simulink model "TosAeb"  (R2021a)
settings: Solver=FixedStepDiscrete  StartTime=0.0  StopTime=inf  FixedStep=0.01  RelTol=1e-3
undefined symbols (not defined in the file or in its folder): ACCModSts, AccSts1, AEB_ObjectMovingOrientation, AEB_ObjectMovingState, CN_BTCActive, CN_CurrentGearR, CN_CurrentGearR_DHT, CN_PTCActive, CN_VDCActive, CN_VSC_EspOff, CN_VSC_FCW_AEB_Error, CN_VSC_FCW_AEB_NoDispay, CN_VSC_FCW_AEB_PerfmDgdtn, CN_VSC_FCW_AEB_TempUnAvlbl, CN_VSC_VehStandstill, CNaccelAvoidMax, CNaccelAvoidMin, CNbrakePedalPress, CNcomfortStrLeftAccelMax, CNcomfortStrLeftAccelMin, CNcomfortStrRightAccelMax, CNcomfortStrRightAccelMin, CNDrvDoorOpen, CNDrvModeEco, CNDrvModeNorm, CNDrvModeOther, CNDrvModeSport, CNDrvSBRreminder, CNNull, CNobjInvalidID, CNPassDoorOpen, CNPassSBRreminder, CNPEBSAccelNegInf, CNPEBSAccelNull, CNPEBSTimeInf, CNPEBSTimeNull, CNSTOPMAX, CNSTOPNULL, CNsubjWidth, CNSwtOFF, CNSwtON, CNTTCMAX, CNTTCNULL, CNVehConfigAEBCycNone, CNVehConfigAEBNone, CNVehConfigAEBPedNone, CNVehConfigFCWNone, CNVehConfigJANone, CollisionSeverity, FALSE, FCWSnvty, JACycObjCalcInfoDefaultParams, JAPedObjCalcInfoDefaultParams, JAVehObjCalcInfoDefaultParams, latCycObjCalcInfoDefaultParams, latPedObjCalcInfoDefaultParams, longObjCalcInfoDefaultParams, ModSts, movingStatus, ObjectType ... (+1112)
libraries: simulink x3031, AEBSFunctionLib x50, Ta_Library x33, AEBSStateMachineLib x23, Mylibrary x12, simulink_extras x7, ObjSelect_lib x3, dspstat3 x2
(part 88 of 102)

== system TosAeb/FcwAebJa_Step_sys/ALG_AEBSystem/ALG_AEBController/AEB_LongTgtInfoCalc/LongObjCalcInfoCalc/AEBS_LongCollisionAssesApp/LongCollisionAvoidAssess/HighLvlBrkCalc/HighLvlBrkCalc4Brake/accelAvoidHighLvlBrkSituation3/If Action Subsystem1/accelAvoidHighLvlBrkCalc/accelAvoidLowLvlBrkCalc/Situation3 ==
  note: aEgo^2
      | aAvoid = ----------------------------------
      | 		vObj^2
      | 		---------  - 2(dRel - dScure)
      | 		aObj
  subjVel : Inport
  vxAbsObj : Inport  Port=2
  axAbsObj : Inport  Port=3
  dxEgoBrake : Inport  Port=4
  function : TriggerPort  TriggerType=function-call  VariantControl=Variant
  Divide : Product  Inputs=*/
  Divide1 : Product  Inputs=*/
  Gain : Gain  Gain=2
  Math Function : Math  Operator=square  SignedPower=on  SaturateOnIntegerOverflow=on
  Math Function1 : Math  Operator=square  SignedPower=on  SaturateOnIntegerOverflow=on
  Subtract : Sum  Inputs=+-
  accelAvoidLowLvlBrk : Outport
  wires:
  vxAbsObj/1 -> Math Function/1
  subjVel/1 -> Math Function1/1
  Math Function/1 -> Divide/1
  Gain/1 -> Subtract/2
  Divide1/1 -> accelAvoidLowLvlBrk/1
  Math Function1/1 -> Divide1/1
  Divide/1 -> Subtract/1
  axAbsObj/1 -> Divide/2
  dxEgoBrake/1 -> Gain/1
  Subtract/1 -> Divide1/2

== system TosAeb/FcwAebJa_Step_sys/ALG_AEBSystem/ALG_AEBController/AEB_LongTgtInfoCalc/LongObjCalcInfoCalc/AEBS_LongCollisionAssesApp/LongCollisionAvoidAssess/HighLvlBrkCalc/HighLvlBrkCalc4Brake/accelAvoidHighLvlBrkSituation3/If Action Subsystem1/accelAvoidHighLvlBrkCalc/accelAvoidLowLvlBrkCalc/Situation4 ==
  function : TriggerPort  TriggerType=function-call  VariantControl=Variant
  Constant : Constant  Value=CNNull  VectorParams1D=off
  accelAvoidLowLvlBrk : Outport
  wires:
  Constant/1 -> accelAvoidLowLvlBrk/1

== system TosAeb/FcwAebJa_Step_sys/ALG_AEBSystem/ALG_AEBController/AEB_LongTgtInfoCalc/LongObjCalcInfoCalc/AEBS_LongCollisionAssesApp/LongCollisionAvoidAssess/HighLvlBrkCalc/HighLvlBrkCalc4Brake/accelAvoidHighLvlBrkSituation3/If Action Subsystem1/accelAvoidHighLvlBrkCalc/accelAvoidLowLvlBrkCalc/accelAvoidLowLvlBrkLimit ==
  accelAvoidLowLvlBrkNoLimit : Inport
  Saturation : Saturate  UpperLimit=CNNull  LowerLimit=CNaccelAvoidMin
      settings: AttributesFormatString=|
      | UpperLimit:%<UpperLimit>
      | LowerLimit:%<LowerLimit>
  accelAvoidLowLvlBrk : Outport
  wires:
  accelAvoidLowLvlBrkNoLimit/1 -> Saturation/1
  Saturation/1 -> accelAvoidLowLvlBrk/1

== system TosAeb/FcwAebJa_Step_sys/ALG_AEBSystem/ALG_AEBController/AEB_LongTgtInfoCalc/LongObjCalcInfoCalc/AEBS_LongCollisionAssesApp/LongCollisionAvoidAssess/HighLvlBrkCalc/HighLvlBrkCalc4Brake/accelAvoidHighLvlBrkSituation3/If Action Subsystem1/accelAvoidHighLvlBrkCalc/accelAvoidLowLvlBrkCalc1/Situation1 ==
  dxEgoBrake : Inport
  vx : Inport  Port=2
  tObjStop : Inport  Port=3
  subjVel : Inport  Port=4
  vxAbsObj : Inport  Port=5
  axAbsObj : Inport  Port=6
  function : TriggerPort  TriggerType=function-call  VariantControl=Variant
  Bus Creator : BusCreator  Inputs=6
  Bus Selector : BusSelector  OutputSignals=dxEgoBrake,vxRelTargetObjCalc,tObjStop
  Bus Selector1 : BusSelector  OutputSignals=axAbsTargetObjCalc,vxRelTargetObjCalc,dxEgoBrake
  Bus Selector2 : BusSelector  OutputSignals=subjVel,vxAbsTargetObjCalc,axAbsTargetObjCalc,dxEgoBrake
  Case1 : Subsystem  TreatAsAtomicUnit=on
  Case2 : Subsystem  TreatAsAtomicUnit=on
  If : If  IfExpression=u1
  Merge : Merge
  collisionFirstFlgCalc : Subsystem
  accelAvoidLowLvlBrk : Outport
  wires:
  collisionFirstFlgCalc/1(collisionFirstFlg) -> If/1
  Bus Selector/3 -> collisionFirstFlgCalc/3(tObjStop)  "<tObjStop>"
  Bus Selector/2 -> collisionFirstFlgCalc/2(vx)  "<vxRelTargetObjCalc>"
  Bus Selector/1 -> collisionFirstFlgCalc/1(dxEgoBrake)  "<dxEgoBrake>"
  Bus Selector2/4 -> Case2/4(dxEgoBrake)  "<dxEgoBrake>"
  Bus Selector2/3 -> Case2/3(axAbsObj)  "<axAbsTargetObjCalc>"
  Bus Selector2/2 -> Case2/2(vxAbsObj)  "<vxAbsTargetObjCalc>"
  Bus Selector2/1 -> Case2/1(subjVel)  "<subjVel>"
  Bus Selector1/3 -> Case1/3(dxEgoBrake)  "<dxEgoBrake>"
  Bus Selector1/2 -> Case1/2(vx)  "<vxRelTargetObjCalc>"
  Bus Selector1/1 -> Case1/1(axAbsObj)  "<axAbsTargetObjCalc>"
  Bus Creator/1 -> Bus Selector/1, Bus Selector1/1, Bus Selector2/1
  vxAbsObj/1 -> Bus Creator/5  "vxAbsTargetObjCalc"
  axAbsObj/1 -> Bus Creator/6  "axAbsTargetObjCalc"
  dxEgoBrake/1 -> Bus Creator/1  "dxEgoBrake"
  subjVel/1 -> Bus Creator/4  "subjVel"
  vx/1 -> Bus Creator/2  "vxRelTargetObjCalc"
  tObjStop/1 -> Bus Creator/3  "tObjStop"
  [CASE2] -> Case2/Action
  If/2 -> [CASE2]
  Case2/1(accelAvoid) -> Merge/2
  Case1/1(accelAvoid) -> Merge/1
  Merge/1 -> accelAvoidLowLvlBrk/1
  If/1 -> Case1/Action

== system TosAeb/FcwAebJa_Step_sys/ALG_AEBSystem/ALG_AEBController/AEB_LongTgtInfoCalc/LongObjCalcInfoCalc/AEBS_LongCollisionAssesApp/LongCollisionAvoidAssess/HighLvlBrkCalc/HighLvlBrkCalc4Brake/accelAvoidHighLvlBrkSituation3/If Action Subsystem1/accelAvoidHighLvlBrkCalc/accelAvoidLowLvlBrkCalc1/Situation2 ==
  axAbsObj : Inport
  vx : Inport  Port=2
  dxEgoBrake : Inport  Port=3
  function : TriggerPort  TriggerType=function-call  VariantControl=Variant
  Divide : Product  Inputs=*/
  Gain : Gain  Gain=2
  Math Function : Math  Operator=square  SignedPower=on  SaturateOnIntegerOverflow=on
  Subtract : Sum  Inputs=+-
  accelAvoidLowLvlBrk : Outport
  wires:
  Divide/1 -> Subtract/2
  Subtract/1 -> accelAvoidLowLvlBrk/1
  Gain/1 -> Divide/2
  dxEgoBrake/1 -> Gain/1
  Math Function/1 -> Divide/1
  vx/1 -> Math Function/1
  axAbsObj/1 -> Subtract/1

== system TosAeb/FcwAebJa_Step_sys/ALG_AEBSystem/ALG_AEBController/AEB_LongTgtInfoCalc/LongObjCalcInfoCalc/AEBS_LongCollisionAssesApp/LongCollisionAvoidAssess/HighLvlBrkCalc/HighLvlBrkCalc4Brake/accelAvoidHighLvlBrkSituation3/If Action Subsystem1/accelAvoidHighLvlBrkCalc/accelAvoidLowLvlBrkCalc1/Situation3 ==
  note: aEgo^2
      | aAvoid = ----------------------------------
      | 		vObj^2
      | 		---------  - 2(dRel - dScure)
      | 		aObj
  subjVel : Inport
  vxAbsObj : Inport  Port=2
  axAbsObj : Inport  Port=3
  dxEgoBrake : Inport  Port=4
  function : TriggerPort  TriggerType=function-call  VariantControl=Variant
  Divide : Product  Inputs=*/
  Divide1 : Product  Inputs=*/
  Gain : Gain  Gain=2
  Math Function : Math  Operator=square  SignedPower=on  SaturateOnIntegerOverflow=on
  Math Function1 : Math  Operator=square  SignedPower=on  SaturateOnIntegerOverflow=on
  Subtract : Sum  Inputs=+-
  accelAvoidLowLvlBrk : Outport
  wires:
  Subtract/1 -> Divide1/2
  dxEgoBrake/1 -> Gain/1
  axAbsObj/1 -> Divide/2
  Divide/1 -> Subtract/1
  Math Function1/1 -> Divide1/1
  Divide1/1 -> accelAvoidLowLvlBrk/1
  Gain/1 -> Subtract/2
  Math Function/1 -> Divide/1
  subjVel/1 -> Math Function1/1
  vxAbsObj/1 -> Math Function/1

== system TosAeb/FcwAebJa_Step_sys/ALG_AEBSystem/ALG_AEBController/AEB_LongTgtInfoCalc/LongObjCalcInfoCalc/AEBS_LongCollisionAssesApp/LongCollisionAvoidAssess/HighLvlBrkCalc/HighLvlBrkCalc4Brake/accelAvoidHighLvlBrkSituation3/If Action Subsystem1/accelAvoidHighLvlBrkCalc/accelAvoidLowLvlBrkCalc1/Situation4 ==
  function : TriggerPort  TriggerType=function-call  VariantControl=Variant
  Constant : Constant  Value=CNaccelAvoidMax  VectorParams1D=off
  accelAvoidLowLvlBrk : Outport
  wires:
  Constant/1 -> accelAvoidLowLvlBrk/1

== system TosAeb/FcwAebJa_Step_sys/ALG_AEBSystem/ALG_AEBController/AEB_LongTgtInfoCalc/LongObjCalcInfoCalc/AEBS_LongCollisionAssesApp/LongCollisionAvoidAssess/HighLvlBrkCalc/HighLvlBrkCalc4Brake/accelAvoidHighLvlBrkSituation3/If Action Subsystem1/accelAvoidHighLvlBrkCalc/accelAvoidLowLvlBrkCalc1/accelAvoidLowLvlBrkLimit ==
  accelAvoidLowLvlBrkNoLimit : Inport
  Saturation : Saturate  UpperLimit=CNaccelAvoidMax  LowerLimit=CNaccelAvoidMin
      settings: AttributesFormatString=|
      | UpperLimit:%<UpperLimit>
      | LowerLimit:%<LowerLimit>
  accelAvoidLowLvlBrk : Outport
  wires:
  Saturation/1 -> accelAvoidLowLvlBrk/1
  accelAvoidLowLvlBrkNoLimit/1 -> Saturation/1

== system TosAeb/FcwAebJa_Step_sys/ALG_AEBSystem/ALG_AEBController/AEB_LongTgtInfoCalc/LongObjCalcInfoCalc/AEBS_LongCollisionAssesApp/LongCollisionAvoidAssess/HighLvlBrkCalc/HighLvlBrkCalc4Warning/accelAvoidHighLvlBrkSituation2/accelAvoidHighLvlBrkCalc/If Action Subsystem1/accelAvoidLowLvlBrkCalc/Situation1 ==
  dxEgoBrake : Inport
  vx : Inport  Port=2
  tObjStop : Inport  Port=3
  subjVel : Inport  Port=4
  vxAbsObj : Inport  Port=5
  axAbsObj : Inport  Port=6
  function : TriggerPort  TriggerType=function-call  VariantControl=Variant
  Bus Creator : BusCreator  Inputs=6
  Bus Selector : BusSelector  OutputSignals=dxEgoBrake,vx,tObjStop
  Bus Selector1 : BusSelector  OutputSignals=axAbsObj,vx,dxEgoBrake
  Bus Selector2 : BusSelector  OutputSignals=subjVel,vxAbsObj,axAbsObj,dxEgoBrake
  Case1 : Subsystem  TreatAsAtomicUnit=on
  Case2 : Subsystem  TreatAsAtomicUnit=on
  If : If  IfExpression=u1
  Merge : Merge
  collisionFirstFlgCalc : Subsystem
  accelAvoidLowLvlBrk : Outport
  wires:
  collisionFirstFlgCalc/1(collisionFirstFlg) -> If/1
  Bus Selector/3 -> collisionFirstFlgCalc/3(tObjStop)  "<tObjStop>"
  Bus Selector/2 -> collisionFirstFlgCalc/2(vx)  "<vx>"
  Bus Selector/1 -> collisionFirstFlgCalc/1(dxEgoBrake)  "<dxEgoBrake>"
  Bus Selector2/4 -> Case2/4(dxEgoBrake)  "<dxEgoBrake>"
  Bus Selector2/3 -> Case2/3(axAbsObj)  "<axAbsObj>"
  Bus Selector2/2 -> Case2/2(vxAbsObj)  "<vxAbsObj>"
  Bus Selector2/1 -> Case2/1(subjVel)  "<subjVel>"
  Bus Selector1/3 -> Case1/3(dxEgoBrake)  "<dxEgoBrake>"
  Bus Selector1/2 -> Case1/2(vx)  "<vx>"
  Bus Selector1/1 -> Case1/1(axAbsObj)  "<axAbsObj>"
  Bus Creator/1 -> Bus Selector/1, Bus Selector1/1, Bus Selector2/1
  vxAbsObj/1 -> Bus Creator/5  "vxAbsObj"
  axAbsObj/1 -> Bus Creator/6  "axAbsObj"
  dxEgoBrake/1 -> Bus Creator/1  "dxEgoBrake"
  subjVel/1 -> Bus Creator/4  "subjVel"
  vx/1 -> Bus Creator/2  "vx"
  tObjStop/1 -> Bus Creator/3  "tObjStop"
  [CASE2] -> Case2/Action
  If/2 -> [CASE2]
  Case2/1(accelAvoid) -> Merge/2
  Case1/1(accelAvoid) -> Merge/1
  Merge/1 -> accelAvoidLowLvlBrk/1
  If/1 -> Case1/Action

== system TosAeb/FcwAebJa_Step_sys/ALG_AEBSystem/ALG_AEBController/AEB_LongTgtInfoCalc/LongObjCalcInfoCalc/AEBS_LongCollisionAssesApp/LongCollisionAvoidAssess/HighLvlBrkCalc/HighLvlBrkCalc4Warning/accelAvoidHighLvlBrkSituation2/accelAvoidHighLvlBrkCalc/If Action Subsystem1/accelAvoidLowLvlBrkCalc/Situation2 ==
  axAbsObj : Inport
  vx : Inport  Port=2
  dxEgoBrake : Inport  Port=3
  function : TriggerPort  TriggerType=function-call  VariantControl=Variant
  Divide : Product  Inputs=*/
  Gain : Gain  Gain=2
  Math Function : Math  Operator=square  SignedPower=on  SaturateOnIntegerOverflow=on
  Subtract : Sum  Inputs=+-
  accelAvoidLowLvlBrk : Outport
  wires:
  Divide/1 -> Subtract/2
  Subtract/1 -> accelAvoidLowLvlBrk/1
  Gain/1 -> Divide/2
  dxEgoBrake/1 -> Gain/1
  Math Function/1 -> Divide/1
  vx/1 -> Math Function/1
  axAbsObj/1 -> Subtract/1

== system TosAeb/FcwAebJa_Step_sys/ALG_AEBSystem/ALG_AEBController/AEB_LongTgtInfoCalc/LongObjCalcInfoCalc/AEBS_LongCollisionAssesApp/LongCollisionAvoidAssess/HighLvlBrkCalc/HighLvlBrkCalc4Warning/accelAvoidHighLvlBrkSituation2/accelAvoidHighLvlBrkCalc/If Action Subsystem1/accelAvoidLowLvlBrkCalc/Situation3 ==
  note: aEgo^2
      | aAvoid = ----------------------------------
      | 		vObj^2
      | 		---------  - 2(dRel - dScure)
      | 		aObj
  subjVel : Inport
  vxAbsObj : Inport  Port=2
  axAbsObj : Inport  Port=3
  dxEgoBrake : Inport  Port=4
  function : TriggerPort  TriggerType=function-call  VariantControl=Variant
  Divide : Product  Inputs=*/
  Divide1 : Product  Inputs=*/
  Gain : Gain  Gain=2
  Math Function : Math  Operator=square  SignedPower=on  SaturateOnIntegerOverflow=on
  Math Function1 : Math  Operator=square  SignedPower=on  SaturateOnIntegerOverflow=on
  Subtract : Sum  Inputs=+-
  accelAvoidLowLvlBrk : Outport
  wires:
  Subtract/1 -> Divide1/2
  dxEgoBrake/1 -> Gain/1
  axAbsObj/1 -> Divide/2
  Divide/1 -> Subtract/1
  Math Function1/1 -> Divide1/1
  Divide1/1 -> accelAvoidLowLvlBrk/1
  Gain/1 -> Subtract/2
  Math Function/1 -> Divide/1
  subjVel/1 -> Math Function1/1
  vxAbsObj/1 -> Math Function/1

== system TosAeb/FcwAebJa_Step_sys/ALG_AEBSystem/ALG_AEBController/AEB_LongTgtInfoCalc/LongObjCalcInfoCalc/AEBS_LongCollisionAssesApp/LongCollisionAvoidAssess/HighLvlBrkCalc/HighLvlBrkCalc4Warning/accelAvoidHighLvlBrkSituation2/accelAvoidHighLvlBrkCalc/If Action Subsystem1/accelAvoidLowLvlBrkCalc/Situation4 ==
  function : TriggerPort  TriggerType=function-call  VariantControl=Variant
  Constant : Constant  Value=CNNull  VectorParams1D=off
  accelAvoidLowLvlBrk : Outport
  wires:
  Constant/1 -> accelAvoidLowLvlBrk/1

== system TosAeb/FcwAebJa_Step_sys/ALG_AEBSystem/ALG_AEBController/AEB_LongTgtInfoCalc/LongObjCalcInfoCalc/AEBS_LongCollisionAssesApp/LongCollisionAvoidAssess/HighLvlBrkCalc/HighLvlBrkCalc4Warning/accelAvoidHighLvlBrkSituation2/accelAvoidHighLvlBrkCalc/If Action Subsystem1/accelAvoidLowLvlBrkCalc/accelAvoidLowLvlBrkLimit ==
  accelAvoidLowLvlBrkNoLimit : Inport
  Saturation : Saturate  UpperLimit=CNNull  LowerLimit=CNaccelAvoidMin
      settings: AttributesFormatString=|
      | UpperLimit:%<UpperLimit>
      | LowerLimit:%<LowerLimit>
  accelAvoidLowLvlBrk : Outport
  wires:
  Saturation/1 -> accelAvoidLowLvlBrk/1
  accelAvoidLowLvlBrkNoLimit/1 -> Saturation/1

== system TosAeb/FcwAebJa_Step_sys/ALG_AEBSystem/ALG_AEBController/AEB_LongTgtInfoCalc/LongObjCalcInfoCalc/AEBS_LongCollisionAssesApp/LongCollisionAvoidAssess/HighLvlBrkCalc/HighLvlBrkCalc4Warning/accelAvoidHighLvlBrkSituation2/accelAvoidHighLvlBrkCalc/If Action Subsystem1/accelAvoidLowLvlBrkCalc1/Situation1 ==
  dxEgoBrake : Inport
  vx : Inport  Port=2
  tObjStop : Inport  Port=3
  subjVel : Inport  Port=4
  vxAbsObj : Inport  Port=5
  axAbsObj : Inport  Port=6
  function : TriggerPort  TriggerType=function-call  VariantControl=Variant
  Bus Creator : BusCreator  Inputs=6
  Bus Selector : BusSelector  OutputSignals=dxEgoBrake,vxRelTargetObjCalc,tObjStop
  Bus Selector1 : BusSelector  OutputSignals=axAbsTargetObjCalc,vxRelTargetObjCalc,dxEgoBrake
  Bus Selector2 : BusSelector  OutputSignals=subjVel,vxAbsTargetObjCalc,axAbsTargetObjCalc,dxEgoBrake
  Case1 : Subsystem  TreatAsAtomicUnit=on
  Case2 : Subsystem  TreatAsAtomicUnit=on
  If : If  IfExpression=u1
  Merge : Merge
  collisionFirstFlgCalc : Subsystem
  accelAvoidLowLvlBrk : Outport
  wires:
  If/1 -> Case1/Action
  Merge/1 -> accelAvoidLowLvlBrk/1
  Case1/1(accelAvoid) -> Merge/1
  Case2/1(accelAvoid) -> Merge/2
  If/2 -> [CASE2]
  [CASE2] -> Case2/Action
  tObjStop/1 -> Bus Creator/3  "tObjStop"
  vx/1 -> Bus Creator/2  "vxRelTargetObjCalc"
  subjVel/1 -> Bus Creator/4  "subjVel"
  dxEgoBrake/1 -> Bus Creator/1  "dxEgoBrake"
  axAbsObj/1 -> Bus Creator/6  "axAbsTargetObjCalc"
  vxAbsObj/1 -> Bus Creator/5  "vxAbsTargetObjCalc"
  Bus Creator/1 -> Bus Selector2/1, Bus Selector1/1, Bus Selector/1
  Bus Selector1/1 -> Case1/1(axAbsObj)  "<axAbsTargetObjCalc>"
  Bus Selector1/2 -> Case1/2(vx)  "<vxRelTargetObjCalc>"
  Bus Selector1/3 -> Case1/3(dxEgoBrake)  "<dxEgoBrake>"
  Bus Selector2/1 -> Case2/1(subjVel)  "<subjVel>"
  Bus Selector2/2 -> Case2/2(vxAbsObj)  "<vxAbsTargetObjCalc>"
  Bus Selector2/3 -> Case2/3(axAbsObj)  "<axAbsTargetObjCalc>"
  Bus Selector2/4 -> Case2/4(dxEgoBrake)  "<dxEgoBrake>"
  Bus Selector/1 -> collisionFirstFlgCalc/1(dxEgoBrake)  "<dxEgoBrake>"
  Bus Selector/2 -> collisionFirstFlgCalc/2(vx)  "<vxRelTargetObjCalc>"
  Bus Selector/3 -> collisionFirstFlgCalc/3(tObjStop)  "<tObjStop>"
  collisionFirstFlgCalc/1(collisionFirstFlg) -> If/1

== system TosAeb/FcwAebJa_Step_sys/ALG_AEBSystem/ALG_AEBController/AEB_LongTgtInfoCalc/LongObjCalcInfoCalc/AEBS_LongCollisionAssesApp/LongCollisionAvoidAssess/HighLvlBrkCalc/HighLvlBrkCalc4Warning/accelAvoidHighLvlBrkSituation2/accelAvoidHighLvlBrkCalc/If Action Subsystem1/accelAvoidLowLvlBrkCalc1/Situation2 ==
  axAbsObj : Inport
  vx : Inport  Port=2
  dxEgoBrake : Inport  Port=3
  function : TriggerPort  TriggerType=function-call  VariantControl=Variant
  Divide : Product  Inputs=*/
  Gain : Gain  Gain=2
  Math Function : Math  Operator=square  SignedPower=on  SaturateOnIntegerOverflow=on
  Subtract : Sum  Inputs=+-
  accelAvoidLowLvlBrk : Outport
  wires:
  axAbsObj/1 -> Subtract/1
  vx/1 -> Math Function/1
  Math Function/1 -> Divide/1
  dxEgoBrake/1 -> Gain/1
  Gain/1 -> Divide/2
  Subtract/1 -> accelAvoidLowLvlBrk/1
  Divide/1 -> Subtract/2

== system TosAeb/FcwAebJa_Step_sys/ALG_AEBSystem/ALG_AEBController/AEB_LongTgtInfoCalc/LongObjCalcInfoCalc/AEBS_LongCollisionAssesApp/LongCollisionAvoidAssess/HighLvlBrkCalc/HighLvlBrkCalc4Warning/accelAvoidHighLvlBrkSituation2/accelAvoidHighLvlBrkCalc/If Action Subsystem1/accelAvoidLowLvlBrkCalc1/Situation3 ==
  note: aEgo^2
      | aAvoid = ----------------------------------
      | 		vObj^2
      | 		---------  - 2(dRel - dScure)
      | 		aObj
  subjVel : Inport
  vxAbsObj : Inport  Port=2
  axAbsObj : Inport  Port=3
  dxEgoBrake : Inport  Port=4
  function : TriggerPort  TriggerType=function-call  VariantControl=Variant
  Divide : Product  Inputs=*/
  Divide1 : Product  Inputs=*/
  Gain : Gain  Gain=2
  Math Function : Math  Operator=square  SignedPower=on  SaturateOnIntegerOverflow=on
  Math Function1 : Math  Operator=square  SignedPower=on  SaturateOnIntegerOverflow=on
  Subtract : Sum  Inputs=+-
  accelAvoidLowLvlBrk : Outport
  wires:
  vxAbsObj/1 -> Math Function/1
  subjVel/1 -> Math Function1/1
  Math Function/1 -> Divide/1
  Gain/1 -> Subtract/2
  Divide1/1 -> accelAvoidLowLvlBrk/1
  Math Function1/1 -> Divide1/1
  Divide/1 -> Subtract/1
  axAbsObj/1 -> Divide/2
  dxEgoBrake/1 -> Gain/1
  Subtract/1 -> Divide1/2

== system TosAeb/FcwAebJa_Step_sys/ALG_AEBSystem/ALG_AEBController/AEB_LongTgtInfoCalc/LongObjCalcInfoCalc/AEBS_LongCollisionAssesApp/LongCollisionAvoidAssess/HighLvlBrkCalc/HighLvlBrkCalc4Warning/accelAvoidHighLvlBrkSituation2/accelAvoidHighLvlBrkCalc/If Action Subsystem1/accelAvoidLowLvlBrkCalc1/Situation4 ==
  function : TriggerPort  TriggerType=function-call  VariantControl=Variant
  Constant : Constant  Value=CNaccelAvoidMax  VectorParams1D=off
  accelAvoidLowLvlBrk : Outport
  wires:
  Constant/1 -> accelAvoidLowLvlBrk/1

== system TosAeb/FcwAebJa_Step_sys/ALG_AEBSystem/ALG_AEBController/AEB_LongTgtInfoCalc/LongObjCalcInfoCalc/AEBS_LongCollisionAssesApp/LongCollisionAvoidAssess/HighLvlBrkCalc/HighLvlBrkCalc4Warning/accelAvoidHighLvlBrkSituation2/accelAvoidHighLvlBrkCalc/If Action Subsystem1/accelAvoidLowLvlBrkCalc1/accelAvoidLowLvlBrkLimit ==
  accelAvoidLowLvlBrkNoLimit : Inport
  Saturation : Saturate  UpperLimit=CNaccelAvoidMax  LowerLimit=CNaccelAvoidMin
      settings: AttributesFormatString=|
      | UpperLimit:%<UpperLimit>
      | LowerLimit:%<LowerLimit>
  accelAvoidLowLvlBrk : Outport
  wires:
  accelAvoidLowLvlBrkNoLimit/1 -> Saturation/1
  Saturation/1 -> accelAvoidLowLvlBrk/1

== system TosAeb/FcwAebJa_Step_sys/ALG_AEBSystem/ALG_AEBController/AEB_LongTgtInfoCalc/LongObjCalcInfoCalc/AEBS_LongCollisionAssesApp/LongCollisionAvoidAssess/HighLvlBrkCalc/HighLvlBrkCalc4Warning/accelAvoidHighLvlBrkSituation3/If Action Subsystem1/accelAvoidHighLvlBrkCalc/accelAvoidLowLvlBrkCalc/Situation1 ==
  dxEgoBrake : Inport
  vx : Inport  Port=2
  tObjStop : Inport  Port=3
  subjVel : Inport  Port=4
  vxAbsObj : Inport  Port=5
  axAbsObj : Inport  Port=6
  function : TriggerPort  TriggerType=function-call  VariantControl=Variant
  Bus Creator : BusCreator  Inputs=6
  Bus Selector : BusSelector  OutputSignals=dxEgoBrake,vx,tObjStop
  Bus Selector1 : BusSelector  OutputSignals=axAbsObj,vx,dxEgoBrake
  Bus Selector2 : BusSelector  OutputSignals=subjVel,vxAbsObj,axAbsObj,dxEgoBrake
  Case1 : Subsystem  TreatAsAtomicUnit=on
  Case2 : Subsystem  TreatAsAtomicUnit=on
  If : If  IfExpression=u1
  Merge : Merge
  collisionFirstFlgCalc : Subsystem
  accelAvoidLowLvlBrk : Outport
  wires:
  collisionFirstFlgCalc/1(collisionFirstFlg) -> If/1
  Bus Selector/3 -> collisionFirstFlgCalc/3(tObjStop)  "<tObjStop>"
  Bus Selector/2 -> collisionFirstFlgCalc/2(vx)  "<vx>"
  Bus Selector/1 -> collisionFirstFlgCalc/1(dxEgoBrake)  "<dxEgoBrake>"
  Bus Selector2/4 -> Case2/4(dxEgoBrake)  "<dxEgoBrake>"
  Bus Selector2/3 -> Case2/3(axAbsObj)  "<axAbsObj>"
  Bus Selector2/2 -> Case2/2(vxAbsObj)  "<vxAbsObj>"
  Bus Selector2/1 -> Case2/1(subjVel)  "<subjVel>"
  Bus Selector1/3 -> Case1/3(dxEgoBrake)  "<dxEgoBrake>"
  Bus Selector1/2 -> Case1/2(vx)  "<vx>"
  Bus Selector1/1 -> Case1/1(axAbsObj)  "<axAbsObj>"
  Bus Creator/1 -> Bus Selector/1, Bus Selector1/1, Bus Selector2/1
  vxAbsObj/1 -> Bus Creator/5  "vxAbsObj"
  axAbsObj/1 -> Bus Creator/6  "axAbsObj"
  dxEgoBrake/1 -> Bus Creator/1  "dxEgoBrake"
  subjVel/1 -> Bus Creator/4  "subjVel"
  vx/1 -> Bus Creator/2  "vx"
  tObjStop/1 -> Bus Creator/3  "tObjStop"
  [CASE2] -> Case2/Action
  If/2 -> [CASE2]
  Case2/1(accelAvoid) -> Merge/2
  Case1/1(accelAvoid) -> Merge/1
  Merge/1 -> accelAvoidLowLvlBrk/1
  If/1 -> Case1/Action

== system TosAeb/FcwAebJa_Step_sys/ALG_AEBSystem/ALG_AEBController/AEB_LongTgtInfoCalc/LongObjCalcInfoCalc/AEBS_LongCollisionAssesApp/LongCollisionAvoidAssess/HighLvlBrkCalc/HighLvlBrkCalc4Warning/accelAvoidHighLvlBrkSituation3/If Action Subsystem1/accelAvoidHighLvlBrkCalc/accelAvoidLowLvlBrkCalc/Situation2 ==
  axAbsObj : Inport
  vx : Inport  Port=2
  dxEgoBrake : Inport  Port=3
  function : TriggerPort  TriggerType=function-call  VariantControl=Variant
  Divide : Product  Inputs=*/
  Gain : Gain  Gain=2
  Math Function : Math  Operator=square  SignedPower=on  SaturateOnIntegerOverflow=on
  Subtract : Sum  Inputs=+-
  accelAvoidLowLvlBrk : Outport
  wires:
  Divide/1 -> Subtract/2
  Subtract/1 -> accelAvoidLowLvlBrk/1
  Gain/1 -> Divide/2
  dxEgoBrake/1 -> Gain/1
  Math Function/1 -> Divide/1
  vx/1 -> Math Function/1
  axAbsObj/1 -> Subtract/1

== system TosAeb/FcwAebJa_Step_sys/ALG_AEBSystem/ALG_AEBController/AEB_LongTgtInfoCalc/LongObjCalcInfoCalc/AEBS_LongCollisionAssesApp/LongCollisionAvoidAssess/HighLvlBrkCalc/HighLvlBrkCalc4Warning/accelAvoidHighLvlBrkSituation3/If Action Subsystem1/accelAvoidHighLvlBrkCalc/accelAvoidLowLvlBrkCalc/Situation3 ==
  note: aEgo^2
      | aAvoid = ----------------------------------
      | 		vObj^2
      | 		---------  - 2(dRel - dScure)
      | 		aObj
  subjVel : Inport
  vxAbsObj : Inport  Port=2
  axAbsObj : Inport  Port=3
  dxEgoBrake : Inport  Port=4
  function : TriggerPort  TriggerType=function-call  VariantControl=Variant
  Divide : Product  Inputs=*/
  Divide1 : Product  Inputs=*/
  Gain : Gain  Gain=2
  Math Function : Math  Operator=square  SignedPower=on  SaturateOnIntegerOverflow=on
  Math Function1 : Math  Operator=square  SignedPower=on  SaturateOnIntegerOverflow=on
  Subtract : Sum  Inputs=+-
  accelAvoidLowLvlBrk : Outport
  wires:
  Subtract/1 -> Divide1/2
  dxEgoBrake/1 -> Gain/1
  axAbsObj/1 -> Divide/2
  Divide/1 -> Subtract/1
  Math Function1/1 -> Divide1/1
  Divide1/1 -> accelAvoidLowLvlBrk/1
  Gain/1 -> Subtract/2
  Math Function/1 -> Divide/1
  subjVel/1 -> Math Function1/1
  vxAbsObj/1 -> Math Function/1

== system TosAeb/FcwAebJa_Step_sys/ALG_AEBSystem/ALG_AEBController/AEB_LongTgtInfoCalc/LongObjCalcInfoCalc/AEBS_LongCollisionAssesApp/LongCollisionAvoidAssess/HighLvlBrkCalc/HighLvlBrkCalc4Warning/accelAvoidHighLvlBrkSituation3/If Action Subsystem1/accelAvoidHighLvlBrkCalc/accelAvoidLowLvlBrkCalc/Situation4 ==
  function : TriggerPort  TriggerType=function-call  VariantControl=Variant
  Constant : Constant  Value=CNNull  VectorParams1D=off
  accelAvoidLowLvlBrk : Outport
  wires:
  Constant/1 -> accelAvoidLowLvlBrk/1

== system TosAeb/FcwAebJa_Step_sys/ALG_AEBSystem/ALG_AEBController/AEB_LongTgtInfoCalc/LongObjCalcInfoCalc/AEBS_LongCollisionAssesApp/LongCollisionAvoidAssess/HighLvlBrkCalc/HighLvlBrkCalc4Warning/accelAvoidHighLvlBrkSituation3/If Action Subsystem1/accelAvoidHighLvlBrkCalc/accelAvoidLowLvlBrkCalc/accelAvoidLowLvlBrkLimit ==
  accelAvoidLowLvlBrkNoLimit : Inport
  Saturation : Saturate  UpperLimit=CNNull  LowerLimit=CNaccelAvoidMin
      settings: AttributesFormatString=|
      | UpperLimit:%<UpperLimit>
      | LowerLimit:%<LowerLimit>
  accelAvoidLowLvlBrk : Outport
  wires:
  Saturation/1 -> accelAvoidLowLvlBrk/1
  accelAvoidLowLvlBrkNoLimit/1 -> Saturation/1

== system TosAeb/FcwAebJa_Step_sys/ALG_AEBSystem/ALG_AEBController/AEB_LongTgtInfoCalc/LongObjCalcInfoCalc/AEBS_LongCollisionAssesApp/LongCollisionAvoidAssess/HighLvlBrkCalc/HighLvlBrkCalc4Warning/accelAvoidHighLvlBrkSituation3/If Action Subsystem1/accelAvoidHighLvlBrkCalc/accelAvoidLowLvlBrkCalc1/Situation1 ==
  dxEgoBrake : Inport
  vx : Inport  Port=2
  tObjStop : Inport  Port=3
  subjVel : Inport  Port=4
  vxAbsObj : Inport  Port=5
  axAbsObj : Inport  Port=6
  function : TriggerPort  TriggerType=function-call  VariantControl=Variant
  Bus Creator : BusCreator  Inputs=6
  Bus Selector : BusSelector  OutputSignals=dxEgoBrake,vxRelTargetObjCalc,tObjStop
  Bus Selector1 : BusSelector  OutputSignals=axAbsTargetObjCalc,vxRelTargetObjCalc,dxEgoBrake
  Bus Selector2 : BusSelector  OutputSignals=subjVel,vxAbsTargetObjCalc,axAbsTargetObjCalc,dxEgoBrake
  Case1 : Subsystem  TreatAsAtomicUnit=on
  Case2 : Subsystem  TreatAsAtomicUnit=on
  If : If  IfExpression=u1
  Merge : Merge
  collisionFirstFlgCalc : Subsystem
  accelAvoidLowLvlBrk : Outport
  wires:
  If/1 -> Case1/Action
  Merge/1 -> accelAvoidLowLvlBrk/1
  Case1/1(accelAvoid) -> Merge/1
  Case2/1(accelAvoid) -> Merge/2
  If/2 -> [CASE2]
  [CASE2] -> Case2/Action
  tObjStop/1 -> Bus Creator/3  "tObjStop"
  vx/1 -> Bus Creator/2  "vxRelTargetObjCalc"
  subjVel/1 -> Bus Creator/4  "subjVel"
  dxEgoBrake/1 -> Bus Creator/1  "dxEgoBrake"
  axAbsObj/1 -> Bus Creator/6  "axAbsTargetObjCalc"
  vxAbsObj/1 -> Bus Creator/5  "vxAbsTargetObjCalc"
  Bus Creator/1 -> Bus Selector2/1, Bus Selector1/1, Bus Selector/1
  Bus Selector1/1 -> Case1/1(axAbsObj)  "<axAbsTargetObjCalc>"
  Bus Selector1/2 -> Case1/2(vx)  "<vxRelTargetObjCalc>"
  Bus Selector1/3 -> Case1/3(dxEgoBrake)  "<dxEgoBrake>"
  Bus Selector2/1 -> Case2/1(subjVel)  "<subjVel>"
  Bus Selector2/2 -> Case2/2(vxAbsObj)  "<vxAbsTargetObjCalc>"
  Bus Selector2/3 -> Case2/3(axAbsObj)  "<axAbsTargetObjCalc>"
  Bus Selector2/4 -> Case2/4(dxEgoBrake)  "<dxEgoBrake>"
  Bus Selector/1 -> collisionFirstFlgCalc/1(dxEgoBrake)  "<dxEgoBrake>"
  Bus Selector/2 -> collisionFirstFlgCalc/2(vx)  "<vxRelTargetObjCalc>"
  Bus Selector/3 -> collisionFirstFlgCalc/3(tObjStop)  "<tObjStop>"
  collisionFirstFlgCalc/1(collisionFirstFlg) -> If/1

== system TosAeb/FcwAebJa_Step_sys/ALG_AEBSystem/ALG_AEBController/AEB_LongTgtInfoCalc/LongObjCalcInfoCalc/AEBS_LongCollisionAssesApp/LongCollisionAvoidAssess/HighLvlBrkCalc/HighLvlBrkCalc4Warning/accelAvoidHighLvlBrkSituation3/If Action Subsystem1/accelAvoidHighLvlBrkCalc/accelAvoidLowLvlBrkCalc1/Situation2 ==
  axAbsObj : Inport
  vx : Inport  Port=2
  dxEgoBrake : Inport  Port=3
  function : TriggerPort  TriggerType=function-call  VariantControl=Variant
  Divide : Product  Inputs=*/
  Gain : Gain  Gain=2
  Math Function : Math  Operator=square  SignedPower=on  SaturateOnIntegerOverflow=on
  Subtract : Sum  Inputs=+-
  accelAvoidLowLvlBrk : Outport
  wires:
  axAbsObj/1 -> Subtract/1
  vx/1 -> Math Function/1
  Math Function/1 -> Divide/1
  dxEgoBrake/1 -> Gain/1
  Gain/1 -> Divide/2
  Subtract/1 -> accelAvoidLowLvlBrk/1
  Divide/1 -> Subtract/2

== system TosAeb/FcwAebJa_Step_sys/ALG_AEBSystem/ALG_AEBController/AEB_LongTgtInfoCalc/LongObjCalcInfoCalc/AEBS_LongCollisionAssesApp/LongCollisionAvoidAssess/HighLvlBrkCalc/HighLvlBrkCalc4Warning/accelAvoidHighLvlBrkSituation3/If Action Subsystem1/accelAvoidHighLvlBrkCalc/accelAvoidLowLvlBrkCalc1/Situation3 ==
  note: aEgo^2
      | aAvoid = ----------------------------------
      | 		vObj^2
      | 		---------  - 2(dRel - dScure)
      | 		aObj
  subjVel : Inport
  vxAbsObj : Inport  Port=2
  axAbsObj : Inport  Port=3
  dxEgoBrake : Inport  Port=4
  function : TriggerPort  TriggerType=function-call  VariantControl=Variant
  Divide : Product  Inputs=*/
  Divide1 : Product  Inputs=*/
  Gain : Gain  Gain=2
  Math Function : Math  Operator=square  SignedPower=on  SaturateOnIntegerOverflow=on
  Math Function1 : Math  Operator=square  SignedPower=on  SaturateOnIntegerOverflow=on
  Subtract : Sum  Inputs=+-
  accelAvoidLowLvlBrk : Outport
  wires:
  vxAbsObj/1 -> Math Function/1
  subjVel/1 -> Math Function1/1
  Math Function/1 -> Divide/1
  Gain/1 -> Subtract/2
  Divide1/1 -> accelAvoidLowLvlBrk/1
  Math Function1/1 -> Divide1/1
  Divide/1 -> Subtract/1
  axAbsObj/1 -> Divide/2
  dxEgoBrake/1 -> Gain/1
  Subtract/1 -> Divide1/2

== system TosAeb/FcwAebJa_Step_sys/ALG_AEBSystem/ALG_AEBController/AEB_LongTgtInfoCalc/LongObjCalcInfoCalc/AEBS_LongCollisionAssesApp/LongCollisionAvoidAssess/HighLvlBrkCalc/HighLvlBrkCalc4Warning/accelAvoidHighLvlBrkSituation3/If Action Subsystem1/accelAvoidHighLvlBrkCalc/accelAvoidLowLvlBrkCalc1/Situation4 ==
  function : TriggerPort  TriggerType=function-call  VariantControl=Variant
  Constant : Constant  Value=CNaccelAvoidMax  VectorParams1D=off
  accelAvoidLowLvlBrk : Outport
  wires:
  Constant/1 -> accelAvoidLowLvlBrk/1

== system TosAeb/FcwAebJa_Step_sys/ALG_AEBSystem/ALG_AEBController/AEB_LongTgtInfoCalc/LongObjCalcInfoCalc/AEBS_LongCollisionAssesApp/LongCollisionAvoidAssess/HighLvlBrkCalc/HighLvlBrkCalc4Warning/accelAvoidHighLvlBrkSituation3/If Action Subsystem1/accelAvoidHighLvlBrkCalc/accelAvoidLowLvlBrkCalc1/accelAvoidLowLvlBrkLimit ==
  accelAvoidLowLvlBrkNoLimit : Inport
  Saturation : Saturate  UpperLimit=CNaccelAvoidMax  LowerLimit=CNaccelAvoidMin
      settings: AttributesFormatString=|
      | UpperLimit:%<UpperLimit>
      | LowerLimit:%<LowerLimit>
  accelAvoidLowLvlBrk : Outport
  wires:
  accelAvoidLowLvlBrkNoLimit/1 -> Saturation/1
  Saturation/1 -> accelAvoidLowLvlBrk/1

== system TosAeb/FcwAebJa_Step_sys/ALG_AEBSystem/ALG_AEBController/AEB_LongTgtInfoCalc/LongObjCalcInfoCalc/AEBS_LongCollisionAssesApp/collisionSeverityHighCalc/collisionSeverityHighCalc1/CollisionAssessHighCalcVersion3/CollisionAssessHighCalc1/CalcBrakePhase1/tEgoStopCalc/tStopCalc/StopTimeFlow/tStopFuncCalc ==
  velRel : Inport
  accelRel : Inport  Port=2
  tStopFuncCalc : TriggerPort  TriggerType=function-call  VariantControl=Variant
      settings: CopyFcn=Stateflow.SLINSF.SimulinkMan.removeBlockPreDeleteFcn  PreDeleteFcn=Stateflow.SLINSF.SimulinkMan.unsafePredeleteFcn
  Abs : Abs
  Abs1 : Abs
  Divide : Product  Inputs=*/
  temptStop : Outport
  wires:
  accelRel/1 -> Abs1/1
  Divide/1 -> temptStop/1
  Abs1/1 -> Divide/2
  Abs/1 -> Divide/1
  velRel/1 -> Abs/1

== system TosAeb/FcwAebJa_Step_sys/ALG_AEBSystem/ALG_AEBController/AEB_LongTgtInfoCalc/LongObjCalcInfoCalc/AEBS_LongCollisionAssesApp/collisionSeverityHighCalc/collisionSeverityHighCalc1/CollisionAssessHighCalcVersion3/CollisionAssessHighCalc1/CalcBrakePhase1/tObjStopCalc/tStopCalc/StopTimeFlow/tStopFuncCalc ==
  velRel : Inport
  accelRel : Inport  Port=2
  tStopFuncCalc : TriggerPort  TriggerType=function-call  VariantControl=Variant
      settings: CopyFcn=Stateflow.SLINSF.SimulinkMan.removeBlockPreDeleteFcn  PreDeleteFcn=Stateflow.SLINSF.SimulinkMan.unsafePredeleteFcn
  Abs : Abs
  Abs1 : Abs
  Divide : Product  Inputs=*/
  temptStop : Outport
  wires:
  accelRel/1 -> Abs1/1
  Divide/1 -> temptStop/1
  Abs1/1 -> Divide/2
  Abs/1 -> Divide/1
  velRel/1 -> Abs/1

== system TosAeb/FcwAebJa_Step_sys/ALG_AEBSystem/ALG_AEBController/AEB_LongTgtInfoCalc/LongObjCalcInfoCalc/AEBS_LongCollisionAssesApp/collisionSeverityHighCalc/collisionSeverityHighCalc1/CollisionAssessHighCalcVersion3/CollisionAssessHighCalc1/CalcBrakePhase1/timeEgovDeltaCalc/timeEgovDelata/StopTimeFlow/tStopFuncCalc ==
  velRel : Inport
  accelRel : Inport  Port=2
  tStopFuncCalc : TriggerPort  TriggerType=function-call  VariantControl=Variant
      settings: CopyFcn=Stateflow.SLINSF.SimulinkMan.removeBlockPreDeleteFcn  PreDeleteFcn=Stateflow.SLINSF.SimulinkMan.unsafePredeleteFcn
  Abs : Abs
  Abs1 : Abs
  Divide : Product  Inputs=*/
  temptStop : Outport
  wires:
  accelRel/1 -> Abs1/1
  Divide/1 -> temptStop/1
  Abs1/1 -> Divide/2
  Abs/1 -> Divide/1
  velRel/1 -> Abs/1

== system TosAeb/FcwAebJa_Step_sys/ALG_AEBSystem/ALG_AEBController/AEB_LongTgtInfoCalc/LongObjCalcInfoCalc/AEBS_LongCollisionAssesApp/collisionSeverityHighCalc/collisionSeverityHighCalc1/CollisionAssessHighCalcVersion3/CollisionAssessHighCalc1/CalcBrakePhase2/tEgoStopCalc/tStopCalc/StopTimeFlow/tStopFuncCalc ==
  velRel : Inport
  accelRel : Inport  Port=2
  tStopFuncCalc : TriggerPort  TriggerType=function-call  VariantControl=Variant
      settings: CopyFcn=Stateflow.SLINSF.SimulinkMan.removeBlockPreDeleteFcn  PreDeleteFcn=Stateflow.SLINSF.SimulinkMan.unsafePredeleteFcn
  Abs : Abs
  Abs1 : Abs
  Divide : Product  Inputs=*/
  temptStop : Outport
  wires:
  accelRel/1 -> Abs1/1
  Divide/1 -> temptStop/1
  Abs1/1 -> Divide/2
  Abs/1 -> Divide/1
  velRel/1 -> Abs/1

== system TosAeb/FcwAebJa_Step_sys/ALG_AEBSystem/ALG_AEBController/AEB_LongTgtInfoCalc/LongObjCalcInfoCalc/AEBS_LongCollisionAssesApp/collisionSeverityHighCalc/collisionSeverityHighCalc1/CollisionAssessHighCalcVersion3/CollisionAssessHighCalc1/CalcBrakePhase2/tObjStopCalc/tStopCalc/StopTimeFlow/tStopFuncCalc ==
  velRel : Inport
  accelRel : Inport  Port=2
  tStopFuncCalc : TriggerPort  TriggerType=function-call  VariantControl=Variant
      settings: CopyFcn=Stateflow.SLINSF.SimulinkMan.removeBlockPreDeleteFcn  PreDeleteFcn=Stateflow.SLINSF.SimulinkMan.unsafePredeleteFcn
  Abs : Abs
  Abs1 : Abs
  Divide : Product  Inputs=*/
  temptStop : Outport
  wires:
  accelRel/1 -> Abs1/1
  Divide/1 -> temptStop/1
  Abs1/1 -> Divide/2
  Abs/1 -> Divide/1
  velRel/1 -> Abs/1

== system TosAeb/FcwAebJa_Step_sys/ALG_AEBSystem/ALG_AEBController/AEB_LongTgtInfoCalc/LongObjCalcInfoCalc/AEBS_LongCollisionAssesApp/collisionSeverityHighCalc/collisionSeverityHighCalc1/CollisionAssessHighCalcVersion3/CollisionAssessHighCalc1/CalcBrakePhase2/timeEgovDeltaCalc/timeEgovDelata/StopTimeFlow/tStopFuncCalc ==
  velRel : Inport
  accelRel : Inport  Port=2
  tStopFuncCalc : TriggerPort  TriggerType=function-call  VariantControl=Variant
      settings: CopyFcn=Stateflow.SLINSF.SimulinkMan.removeBlockPreDeleteFcn  PreDeleteFcn=Stateflow.SLINSF.SimulinkMan.unsafePredeleteFcn
  Abs : Abs
  Abs1 : Abs
  Divide : Product  Inputs=*/
  temptStop : Outport
  wires:
  accelRel/1 -> Abs1/1
  Divide/1 -> temptStop/1
  Abs1/1 -> Divide/2
  Abs/1 -> Divide/1
  velRel/1 -> Abs/1

== system TosAeb/FcwAebJa_Step_sys/ALG_AEBSystem/ALG_AEBController/AEB_LongTgtInfoCalc/LongObjCalcInfoCalc/AEBS_LongCollisionAssesApp/collisionSeverityHighCalc/collisionSeverityHighCalc1/CollisionAssessHighCalcVersion3/CollisionAssessHighCalc1/CalcBrakePhase3/BrkSysRuntime/a0ObjGreatSituation/Subsystem ==
  vtEgo : Inport
  v0Obj : Inport  Port=2
  Relational Operator : RelationalOperator  Operator=>  OutDataTypeStr=boolean  RndMeth=Simplest
  Flg : Outport
  wires:
  vtEgo/1 -> Relational Operator/1
  v0Obj/1 -> Relational Operator/2
  Relational Operator/1 -> Flg/1

== system TosAeb/FcwAebJa_Step_sys/ALG_AEBSystem/ALG_AEBController/AEB_LongTgtInfoCalc/LongObjCalcInfoCalc/AEBS_LongCollisionAssesApp/collisionSeverityHighCalc/collisionSeverityHighCalc1/CollisionAssessHighCalcVersion3/CollisionAssessHighCalc1/CalcBrakePhase3/BrkSysRuntime/a0ObjGreatSituation/vtEgoGreatSituation ==
  Action Port : ActionPort  ActionPortLabel=else
  Constant : Constant  Value=0
  tTime : Outport
  wires:
  Constant/1 -> tTime/1

== system TosAeb/FcwAebJa_Step_sys/ALG_AEBSystem/ALG_AEBController/AEB_LongTgtInfoCalc/LongObjCalcInfoCalc/AEBS_LongCollisionAssesApp/collisionSeverityHighCalc/collisionSeverityHighCalc1/CollisionAssessHighCalcVersion3/CollisionAssessHighCalc1/CalcBrakePhase3/BrkSysRuntime/a0ObjGreatSituation/vtEgoLessSituation ==
  v0Obj : Inport
  vtEgo : Inport  Port=2
  accelMaxDec : Inport  Port=3
  a0Obj : Inport  Port=4
  Action Port : ActionPort  ActionPortLabel=if(u1)
  Divide : Product  Inputs=*/
  Subtract : Sum  Inputs=+-
  Subtract1 : Sum  Inputs=+-
  Unary Minus : UnaryMinus
  tTime : Outport
  wires:
  vtEgo/1 -> Subtract/1
  v0Obj/1 -> Subtract/2
  accelMaxDec/1 -> Subtract1/1
  a0Obj/1 -> Subtract1/2
  Subtract/1 -> Divide/1
  Subtract1/1 -> Unary Minus/1
  Unary Minus/1 -> Divide/2
  Divide/1 -> tTime/1

== system TosAeb/FcwAebJa_Step_sys/ALG_AEBSystem/ALG_AEBController/AEB_LongTgtInfoCalc/LongObjCalcInfoCalc/AEBS_LongCollisionAssesApp/collisionSeverityHighCalc/collisionSeverityHighCalc1/CollisionAssessHighCalcVersion3/CollisionAssessHighCalc1/CalcBrakePhase3/tEgoStopCalc/tStopCalc/StopTimeFlow/tStopFuncCalc ==
  velRel : Inport
  accelRel : Inport  Port=2
  tStopFuncCalc : TriggerPort  TriggerType=function-call  VariantControl=Variant
      settings: CopyFcn=Stateflow.SLINSF.SimulinkMan.removeBlockPreDeleteFcn  PreDeleteFcn=Stateflow.SLINSF.SimulinkMan.unsafePredeleteFcn
  Abs : Abs
  Abs1 : Abs
  Divide : Product  Inputs=*/
  temptStop : Outport
  wires:
  accelRel/1 -> Abs1/1
  Divide/1 -> temptStop/1
  Abs1/1 -> Divide/2
  Abs/1 -> Divide/1
  velRel/1 -> Abs/1

== system TosAeb/FcwAebJa_Step_sys/ALG_AEBSystem/ALG_AEBController/AEB_LongTgtInfoCalc/LongObjCalcInfoCalc/AEBS_LongCollisionAssesApp/collisionSeverityHighCalc/collisionSeverityHighCalc1/CollisionAssessHighCalcVersion3/CollisionAssessHighCalc1/CalcBrakePhase3/tObjStopCalc/tStopCalc/StopTimeFlow/tStopFuncCalc ==
  velRel : Inport
  accelRel : Inport  Port=2
  tStopFuncCalc : TriggerPort  TriggerType=function-call  VariantControl=Variant
      settings: CopyFcn=Stateflow.SLINSF.SimulinkMan.removeBlockPreDeleteFcn  PreDeleteFcn=Stateflow.SLINSF.SimulinkMan.unsafePredeleteFcn
  Abs : Abs
  Abs1 : Abs
  Divide : Product  Inputs=*/
  temptStop : Outport
  wires:
  accelRel/1 -> Abs1/1
  Divide/1 -> temptStop/1
  Abs1/1 -> Divide/2
  Abs/1 -> Divide/1
  velRel/1 -> Abs/1

== system TosAeb/FcwAebJa_Step_sys/ALG_AEBSystem/ALG_AEBController/AEB_LongTgtInfoCalc/LongObjCalcInfoCalc/AEBS_LongCollisionAssesApp/collisionSeverityHighCalc/collisionSeverityHighCalc1/CollisionAssessHighCalcVersion3/CollisionAssessHighCalc1/CalcBrakePhase3/timeEgovDeltaCalc/timeEgovDelata/StopTimeFlow/tStopFuncCalc ==
  velRel : Inport
  accelRel : Inport  Port=2
  tStopFuncCalc : TriggerPort  TriggerType=function-call  VariantControl=Variant
      settings: CopyFcn=Stateflow.SLINSF.SimulinkMan.removeBlockPreDeleteFcn  PreDeleteFcn=Stateflow.SLINSF.SimulinkMan.unsafePredeleteFcn
  Abs : Abs
  Abs1 : Abs
  Divide : Product  Inputs=*/
  temptStop : Outport
  wires:
  accelRel/1 -> Abs1/1
  Divide/1 -> temptStop/1
  Abs1/1 -> Divide/2
  Abs/1 -> Divide/1
  velRel/1 -> Abs/1

== system TosAeb/FcwAebJa_Step_sys/ALG_AEBSystem/ALG_AEBController/AEB_LongTgtInfoCalc/LongObjCalcInfoCalc/AEBS_LongCollisionAssesApp/collisionSeverityHighCalc/collisionSeverityHighCalc1/CollisionAssessHighCalcVersion3/CollisionAssessHighCalc2/CalcBrakePhase1/tEgoStopCalc/tStopCalc/StopTimeFlow/tStopFuncCalc ==
  velRel : Inport
  accelRel : Inport  Port=2
  tStopFuncCalc : TriggerPort  TriggerType=function-call  VariantControl=Variant
      settings: CopyFcn=Stateflow.SLINSF.SimulinkMan.removeBlockPreDeleteFcn  PreDeleteFcn=Stateflow.SLINSF.SimulinkMan.unsafePredeleteFcn
  Abs : Abs
  Abs1 : Abs
  Divide : Product  Inputs=*/
  temptStop : Outport
  wires:
  accelRel/1 -> Abs1/1
  Divide/1 -> temptStop/1
  Abs1/1 -> Divide/2
  Abs/1 -> Divide/1
  velRel/1 -> Abs/1

== system TosAeb/FcwAebJa_Step_sys/ALG_AEBSystem/ALG_AEBController/AEB_LongTgtInfoCalc/LongObjCalcInfoCalc/AEBS_LongCollisionAssesApp/collisionSeverityHighCalc/collisionSeverityHighCalc1/CollisionAssessHighCalcVersion3/CollisionAssessHighCalc2/CalcBrakePhase1/tObjStopCalc/tStopCalc/StopTimeFlow/tStopFuncCalc ==
  velRel : Inport
  accelRel : Inport  Port=2
  tStopFuncCalc : TriggerPort  TriggerType=function-call  VariantControl=Variant
      settings: CopyFcn=Stateflow.SLINSF.SimulinkMan.removeBlockPreDeleteFcn  PreDeleteFcn=Stateflow.SLINSF.SimulinkMan.unsafePredeleteFcn
  Abs : Abs
  Abs1 : Abs
  Divide : Product  Inputs=*/
  temptStop : Outport
  wires:
  accelRel/1 -> Abs1/1
  Divide/1 -> temptStop/1
  Abs1/1 -> Divide/2
  Abs/1 -> Divide/1
  velRel/1 -> Abs/1

== system TosAeb/FcwAebJa_Step_sys/ALG_AEBSystem/ALG_AEBController/AEB_LongTgtInfoCalc/LongObjCalcInfoCalc/AEBS_LongCollisionAssesApp/collisionSeverityHighCalc/collisionSeverityHighCalc1/CollisionAssessHighCalcVersion3/CollisionAssessHighCalc2/CalcBrakePhase1/timeEgovDelataCalc/timeEgovDelata/StopTimeFlow/tStopFuncCalc ==
  velRel : Inport
  accelRel : Inport  Port=2
  tStopFuncCalc : TriggerPort  TriggerType=function-call  VariantControl=Variant
      settings: CopyFcn=Stateflow.SLINSF.SimulinkMan.removeBlockPreDeleteFcn  PreDeleteFcn=Stateflow.SLINSF.SimulinkMan.unsafePredeleteFcn
  Abs : Abs
  Abs1 : Abs
  Divide : Product  Inputs=*/
  temptStop : Outport
  wires:
  accelRel/1 -> Abs1/1
  Divide/1 -> temptStop/1
  Abs1/1 -> Divide/2
  Abs/1 -> Divide/1
  velRel/1 -> Abs/1

== system TosAeb/FcwAebJa_Step_sys/ALG_AEBSystem/ALG_AEBController/AEB_LongTgtInfoCalc/LongObjCalcInfoCalc/AEBS_LongCollisionAssesApp/collisionSeverityHighCalc/collisionSeverityHighCalc1/CollisionAssessHighCalcVersion3/CollisionAssessHighCalc2/CalcBrakePhase2/tEgoStopCalc/tStopCalc/StopTimeFlow/tStopFuncCalc ==
  velRel : Inport
  accelRel : Inport  Port=2
  tStopFuncCalc : TriggerPort  TriggerType=function-call  VariantControl=Variant
      settings: CopyFcn=Stateflow.SLINSF.SimulinkMan.removeBlockPreDeleteFcn  PreDeleteFcn=Stateflow.SLINSF.SimulinkMan.unsafePredeleteFcn
  Abs : Abs
  Abs1 : Abs
  Divide : Product  Inputs=*/
  temptStop : Outport
  wires:
  accelRel/1 -> Abs1/1
  Divide/1 -> temptStop/1
  Abs1/1 -> Divide/2
  Abs/1 -> Divide/1
  velRel/1 -> Abs/1

== system TosAeb/FcwAebJa_Step_sys/ALG_AEBSystem/ALG_AEBController/AEB_LongTgtInfoCalc/LongObjCalcInfoCalc/AEBS_LongCollisionAssesApp/collisionSeverityHighCalc/collisionSeverityHighCalc1/CollisionAssessHighCalcVersion3/CollisionAssessHighCalc2/CalcBrakePhase2/tObjStopCalc/tStopCalc/StopTimeFlow/tStopFuncCalc ==
  velRel : Inport
  accelRel : Inport  Port=2
  tStopFuncCalc : TriggerPort  TriggerType=function-call  VariantControl=Variant
      settings: CopyFcn=Stateflow.SLINSF.SimulinkMan.removeBlockPreDeleteFcn  PreDeleteFcn=Stateflow.SLINSF.SimulinkMan.unsafePredeleteFcn
  Abs : Abs
  Abs1 : Abs
  Divide : Product  Inputs=*/
  temptStop : Outport
  wires:
  accelRel/1 -> Abs1/1
  Divide/1 -> temptStop/1
  Abs1/1 -> Divide/2
  Abs/1 -> Divide/1
  velRel/1 -> Abs/1

== system TosAeb/FcwAebJa_Step_sys/ALG_AEBSystem/ALG_AEBController/AEB_LongTgtInfoCalc/LongObjCalcInfoCalc/AEBS_LongCollisionAssesApp/collisionSeverityHighCalc/collisionSeverityHighCalc1/CollisionAssessHighCalcVersion3/CollisionAssessHighCalc2/CalcBrakePhase2/timeEgovDelataCalc/timeEgovDelata/StopTimeFlow/tStopFuncCalc ==
  velRel : Inport
  accelRel : Inport  Port=2
  tStopFuncCalc : TriggerPort  TriggerType=function-call  VariantControl=Variant
      settings: CopyFcn=Stateflow.SLINSF.SimulinkMan.removeBlockPreDeleteFcn  PreDeleteFcn=Stateflow.SLINSF.SimulinkMan.unsafePredeleteFcn
  Abs : Abs
  Abs1 : Abs
  Divide : Product  Inputs=*/
  temptStop : Outport
  wires:
  accelRel/1 -> Abs1/1
  Divide/1 -> temptStop/1
  Abs1/1 -> Divide/2
  Abs/1 -> Divide/1
  velRel/1 -> Abs/1

== system TosAeb/FcwAebJa_Step_sys/ALG_AEBSystem/ALG_AEBController/AEB_LongTgtInfoCalc/LongObjCalcInfoCalc/AEBS_LongCollisionAssesApp/collisionSeverityHighCalc/collisionSeverityHighCalc1/CollisionAssessHighCalcVersion3/CollisionAssessHighCalc2/CalcBrakePhase3/BrkSysRuntime/a0ObjGreatSituation/Subsystem ==
  vtEgo : Inport
  v0Obj : Inport  Port=2
  Relational Operator : RelationalOperator  Operator=>  OutDataTypeStr=boolean  RndMeth=Simplest
  Flg : Outport
  wires:
  vtEgo/1 -> Relational Operator/1
  v0Obj/1 -> Relational Operator/2
  Relational Operator/1 -> Flg/1

== system TosAeb/FcwAebJa_Step_sys/ALG_AEBSystem/ALG_AEBController/AEB_LongTgtInfoCalc/LongObjCalcInfoCalc/AEBS_LongCollisionAssesApp/collisionSeverityHighCalc/collisionSeverityHighCalc1/CollisionAssessHighCalcVersion3/CollisionAssessHighCalc2/CalcBrakePhase3/BrkSysRuntime/a0ObjGreatSituation/vtEgoGreatSituation ==
  Action Port : ActionPort
  Constant : Constant  Value=0
  tTime : Outport
  wires:
  Constant/1 -> tTime/1

== system TosAeb/FcwAebJa_Step_sys/ALG_AEBSystem/ALG_AEBController/AEB_LongTgtInfoCalc/LongObjCalcInfoCalc/AEBS_LongCollisionAssesApp/collisionSeverityHighCalc/collisionSeverityHighCalc1/CollisionAssessHighCalcVersion3/CollisionAssessHighCalc2/CalcBrakePhase3/BrkSysRuntime/a0ObjGreatSituation/vtEgoLessSituation ==
  v0Obj : Inport
  vtEgo : Inport  Port=2
  accelMaxDec : Inport  Port=3
  a0Obj : Inport  Port=4
  Action Port : ActionPort
  Divide : Product  Inputs=*/
  Subtract : Sum  Inputs=+-
  Subtract1 : Sum  Inputs=+-
  Unary Minus : UnaryMinus
  tTime : Outport
  wires:
  vtEgo/1 -> Subtract/1
  v0Obj/1 -> Subtract/2
  accelMaxDec/1 -> Subtract1/1
  a0Obj/1 -> Subtract1/2
  Subtract/1 -> Divide/1
  Subtract1/1 -> Unary Minus/1
  Unary Minus/1 -> Divide/2
  Divide/1 -> tTime/1

== system TosAeb/FcwAebJa_Step_sys/ALG_AEBSystem/ALG_AEBController/AEB_LongTgtInfoCalc/LongObjCalcInfoCalc/AEBS_LongCollisionAssesApp/collisionSeverityHighCalc/collisionSeverityHighCalc1/CollisionAssessHighCalcVersion3/CollisionAssessHighCalc2/CalcBrakePhase3/tEgoStopCalc/tStopCalc/StopTimeFlow/tStopFuncCalc ==
  velRel : Inport
  accelRel : Inport  Port=2
  tStopFuncCalc : TriggerPort  TriggerType=function-call  VariantControl=Variant
      settings: CopyFcn=Stateflow.SLINSF.SimulinkMan.removeBlockPreDeleteFcn  PreDeleteFcn=Stateflow.SLINSF.SimulinkMan.unsafePredeleteFcn
  Abs : Abs
  Abs1 : Abs
  Divide : Product  Inputs=*/
  temptStop : Outport
  wires:
  accelRel/1 -> Abs1/1
  Divide/1 -> temptStop/1
  Abs1/1 -> Divide/2
  Abs/1 -> Divide/1
  velRel/1 -> Abs/1

== system TosAeb/FcwAebJa_Step_sys/ALG_AEBSystem/ALG_AEBController/AEB_LongTgtInfoCalc/LongObjCalcInfoCalc/AEBS_LongCollisionAssesApp/collisionSeverityHighCalc/collisionSeverityHighCalc1/CollisionAssessHighCalcVersion3/CollisionAssessHighCalc2/CalcBrakePhase3/tObjStopCalc/tStopCalc/StopTimeFlow/tStopFuncCalc ==
  velRel : Inport
  accelRel : Inport  Port=2
  tStopFuncCalc : TriggerPort  TriggerType=function-call  VariantControl=Variant
      settings: CopyFcn=Stateflow.SLINSF.SimulinkMan.removeBlockPreDeleteFcn  PreDeleteFcn=Stateflow.SLINSF.SimulinkMan.unsafePredeleteFcn
  Abs : Abs
  Abs1 : Abs
  Divide : Product  Inputs=*/
  temptStop : Outport
  wires:
  accelRel/1 -> Abs1/1
  Divide/1 -> temptStop/1
  Abs1/1 -> Divide/2
  Abs/1 -> Divide/1
  velRel/1 -> Abs/1

== system TosAeb/FcwAebJa_Step_sys/ALG_AEBSystem/ALG_AEBController/AEB_LongTgtInfoCalc/LongObjCalcInfoCalc/AEBS_LongCollisionAssesApp/collisionSeverityHighCalc/collisionSeverityHighCalc1/CollisionAssessHighCalcVersion3/CollisionAssessHighCalc2/CalcBrakePhase3/timeEgovDelataCalc/timeEgovDelata/StopTimeFlow/tStopFuncCalc ==
  velRel : Inport
  accelRel : Inport  Port=2
  tStopFuncCalc : TriggerPort  TriggerType=function-call  VariantControl=Variant
      settings: CopyFcn=Stateflow.SLINSF.SimulinkMan.removeBlockPreDeleteFcn  PreDeleteFcn=Stateflow.SLINSF.SimulinkMan.unsafePredeleteFcn
  Abs : Abs
  Abs1 : Abs
  Divide : Product  Inputs=*/
  temptStop : Outport
  wires:
  accelRel/1 -> Abs1/1
  Divide/1 -> temptStop/1
  Abs1/1 -> Divide/2
  Abs/1 -> Divide/1
  velRel/1 -> Abs/1

== system TosAeb/AEB_Objsel/TA_Processor/AEB_ObjSelect/TargetInfoCheck/Data_precess/TA_EffectCalculate/Obj_FilterFlgCalculate/Long_ObjFilter/OverlapCalculate/BoudingBoxCalculate_Else/PosnatTTI/PredictHostPositionAtTTR/CalculateHeading/ExtractVelocitiesAtStartOfSegments/CheckSpeed ==
  note: 0.2m/s
  x : Inport
  y : Inport  Port=2
  LatSpdThresholdNonVeh1 : Constant  Value=SpdThdInAsyEvlrCritEve
  Product : Product
  Product1 : Product
  Product2 : Product
  Relational Operator : RelationalOperator  Operator=>  OutDataTypeStr=boolean  RndMeth=Nearest
  Subtract : Sum
  SpeedValid : Outport
  wires:
  y/1 -> Product/1, Product/2
  Subtract/1 -> Relational Operator/1  "VelocitySquared"
  Product1/1 -> Subtract/1
  Product/1 -> Subtract/2
  x/1 -> Product1/2, Product1/1
  LatSpdThresholdNonVeh1/1 -> Product2/1, Product2/2
  Product2/1 -> Relational Operator/2
  Relational Operator/1 -> SpeedValid/1  "SpeedValid"
